simulink model "dds_cwg_32k"  (R2016b) (continued)
== system dds_cwg_32k/DDC_bu == (continued)
      mask help: FIR filter which can handle multiple time samples in parallel and decimates down to 1 time sample. If coefficients are symmetric, will automatically fold before multiplying.
      mask init:
      | dec_fir_async_init(gcb);
      settings: AttributesFormatString=|
      | 160 taps
      | 18_17 r/i
      mask TypeOptions quantization: Truncate | Round  (unbiased: +/- Inf) | Round  (unbiased: Even Values)
      mask TypeOptions adder_imp: Behavioral | Fabric | DSP48
      mask Callback bus_input:
      | onoff = get_param(gcb, 'bus_input');
      | set_param_state(gcb, 'input_width', onoff)
      | set_param_state(gcb, 'input_bp', onoff)
      | set_param_state(gcb, 'input_type', onoff)
      mask TypeOptions input_type: Signed | Unsigned
  edge_detect2 : Subsystem  AncestorBlock=casper_library_misc/edge_detect  mask(edge_detect){edge=Rising, polarity=Active High, x_in=[0 0.08 0.08 0.16 0.16 0.24 0.24 0.32 0.32 0.4], y_in=[-1 -1  1  1  1  1  1  1  1  1], x_out=[0.6 0.68 0.68 0.76 0.76 0.84 0.84 0.92 0.92 1], y_out=[-1 -1  1  1 -1 -1 -1 -1 -1 -1]}
      mask prompts: edge "Edge Type"; polarity "Output Polarity"; x_in "Input X Positions"; y_in "Input Y Positions"; x_out "Output X Positions"; y_out "Output Y Positions"
      mask help: Output is active for one-cycle after each edge of the specified type (i.e. rising, falling or both).
      mask init: same as dds_cwg_32k/edge_detect12
      settings: AttributesFormatString=|
      | rising edges
      | active high
      mask Help: Output is active for one-cycle after each edge of the specified type (i.e. rising, falling, or both).  Latency is zero.
      mask TypeOptions edge: Rising | Falling | Both
      mask TypeOptions polarity: Active High | Active Low
      mask Display:
      | plot(x_in_num,0.5+y_in_num*0.2);
      | plot(x_out_num,0.5+y_out_num*0.2);
  edge_detect3 : Subsystem  AncestorBlock=casper_library_misc/edge_detect  mask(edge_detect){edge=Rising, polarity=Active High, x_in=[0 0.08 0.08 0.16 0.16 0.24 0.24 0.32 0.32 0.4], y_in=[-1 -1  1  1  1  1  1  1  1  1], x_out=[0.6 0.68 0.68 0.76 0.76 0.84 0.84 0.92 0.92 1], y_out=[-1 -1  1  1 -1 -1 -1 -1 -1 -1]}
      mask prompts: edge "Edge Type"; polarity "Output Polarity"; x_in "Input X Positions"; y_in "Input Y Positions"; x_out "Output X Positions"; y_out "Output Y Positions"
      mask help: Output is active for one-cycle after each edge of the specified type (i.e. rising, falling or both).
      mask init: same as dds_cwg_32k/edge_detect12
      settings: AttributesFormatString=|
      | rising edges
      | active high
      mask Help: Output is active for one-cycle after each edge of the specified type (i.e. rising, falling, or both).  Latency is zero.
      mask TypeOptions edge: Rising | Falling | Both
      mask TypeOptions polarity: Active High | Active Low
      mask Display:
      | plot(x_in_num,0.5+y_in_num*0.2);
      | plot(x_out_num,0.5+y_out_num*0.2);
  mix_sync_cnt : Subsystem  Tag=xps:sw_reg  AncestorBlock=xps_library/Memory/software_register  mask(swreg){io_dir=To Processor, io_delay=0, init_val=0, sample_period=1, names=reg, bitwidths=8, bin_pts=0, arith_types=0, sim_port=off, show_format=on}
      mask prompts: io_dir "I/O direction"; io_delay "I/O delay"; init_val "Initial Value"; sample_period "Sample period"; names "Bitfield names [msb...lsb]"; bitwidths "Bitfield widths"; bin_pts "Bitfield binary pts"; arith_types "Bitfield types, ufix=0, fix=1, bool=2"; sim_port "Provide sim input/output?"; show_format "Print format string?"
      mask help: A 32-bit software-accessible register. Can be divided into bitfields of varying widths and types using the fields in the block mask.
      mask init:
      | swreg_init(gcb)
      settings: AttributesFormatString=1output:uf8.0 = 8 bits
      mask TypeOptions io_dir: To Processor | From Processor
      mask Callback io_dir:
      | mask_enables = get_param(gcb, 'MaskEnables');
      | mask_names = get_param(gcb, 'MaskNames');
      | if strcmp(get_param(gcb, 'io_dir'), 'To Processor'),
      |     mask_enables{ismember(mask_names, 'sample_period')} = 'off';
      | else
      |     mask_enables{ismember(mask_names, 'sample_period')} = 'on';
      | end
      | set_param(gcb, 'MaskEnables', mask_enables);
      mask DialogControl ParameterTabVar0 Prompt: Setup
  pipeline1 : Subsystem  AncestorBlock=casper_library_delays/pipeline  mask(pipeline){latency=2}
      mask help:
      | An explicitly laid-out delay line to avoid mapping to SRL16s
      | for use in pipelining to help achieve timing closure.
      mask init:
      | pipeline_init(gcb,'latency',latency);
      settings: AttributesFormatString=Latency = %<latency>
      mask Display:
      | port_label('input', 1, 'd');
      | port_label('output', 1, 'q');
      | disp(['Z^-', num2str(latency)]);
  pipeline10 : Subsystem  AncestorBlock=casper_library_delays/pipeline  mask(pipeline){latency=2}
      mask help:
      | An explicitly laid-out delay line to avoid mapping to SRL16s
      | for use in pipelining to help achieve timing closure.
      mask init:
      | pipeline_init(gcb,'latency',latency);
      settings: AttributesFormatString=Latency = %<latency>
      mask Display:
      | port_label('input', 1, 'd');
      | port_label('output', 1, 'q');
      | disp(['Z^-', num2str(latency)]);
  pipeline11 : Subsystem  AncestorBlock=casper_library_delays/pipeline  mask(pipeline){latency=2}
      mask help:
      | An explicitly laid-out delay line to avoid mapping to SRL16s
      | for use in pipelining to help achieve timing closure.
      mask init:
      | pipeline_init(gcb,'latency',latency);
      settings: AttributesFormatString=Latency = %<latency>
      mask Display:
      | port_label('input', 1, 'd');
      | port_label('output', 1, 'q');
      | disp(['Z^-', num2str(latency)]);
  pipeline12 : Subsystem  AncestorBlock=casper_library_delays/pipeline  mask(pipeline){latency=2}
      mask help:
      | An explicitly laid-out delay line to avoid mapping to SRL16s
      | for use in pipelining to help achieve timing closure.
      mask init:
      | pipeline_init(gcb,'latency',latency);
      settings: AttributesFormatString=Latency = %<latency>
      mask Display:
      | port_label('input', 1, 'd');
      | port_label('output', 1, 'q');
      | disp(['Z^-', num2str(latency)]);
  pipeline13 : Subsystem  AncestorBlock=casper_library_delays/pipeline  mask(pipeline){latency=2}
      mask help:
      | An explicitly laid-out delay line to avoid mapping to SRL16s
      | for use in pipelining to help achieve timing closure.
      mask init:
      | pipeline_init(gcb,'latency',latency);
      settings: AttributesFormatString=Latency = %<latency>
      mask Display:
      | port_label('input', 1, 'd');
      | port_label('output', 1, 'q');
      | disp(['Z^-', num2str(latency)]);
  pipeline14 : Subsystem  AncestorBlock=casper_library_delays/pipeline  mask(pipeline){latency=2}
      mask help:
      | An explicitly laid-out delay line to avoid mapping to SRL16s
      | for use in pipelining to help achieve timing closure.
      mask init:
      | pipeline_init(gcb,'latency',latency);
      settings: AttributesFormatString=Latency = %<latency>
      mask Display:
      | port_label('input', 1, 'd');
      | port_label('output', 1, 'q');
      | disp(['Z^-', num2str(latency)]);
  pipeline15 : Subsystem  AncestorBlock=casper_library_delays/pipeline  mask(pipeline){latency=2}
      mask help:
      | An explicitly laid-out delay line to avoid mapping to SRL16s
      | for use in pipelining to help achieve timing closure.
      mask init:
      | pipeline_init(gcb,'latency',latency);
      settings: AttributesFormatString=Latency = %<latency>
      mask Display:
      | port_label('input', 1, 'd');
      | port_label('output', 1, 'q');
      | disp(['Z^-', num2str(latency)]);
  pipeline16 : Subsystem  AncestorBlock=casper_library_delays/pipeline  mask(pipeline){latency=2}
      mask help:
      | An explicitly laid-out delay line to avoid mapping to SRL16s
      | for use in pipelining to help achieve timing closure.
      mask init:
      | pipeline_init(gcb,'latency',latency);
      settings: AttributesFormatString=Latency = %<latency>
      mask Display:
      | port_label('input', 1, 'd');
      | port_label('output', 1, 'q');
      | disp(['Z^-', num2str(latency)]);
  pipeline17 : Subsystem  AncestorBlock=casper_library_delays/pipeline  mask(pipeline){latency=2}
      mask help:
      | An explicitly laid-out delay line to avoid mapping to SRL16s
      | for use in pipelining to help achieve timing closure.
      mask init:
      | pipeline_init(gcb,'latency',latency);
      settings: AttributesFormatString=Latency = %<latency>
      mask Display:
      | port_label('input', 1, 'd');
      | port_label('output', 1, 'q');
      | disp(['Z^-', num2str(latency)]);
  pipeline18 : Subsystem  Commented=on  AncestorBlock=casper_library_delays/pipeline  mask(pipeline){latency=2}
      mask help:
      | An explicitly laid-out delay line to avoid mapping to SRL16s
      | for use in pipelining to help achieve timing closure.
      mask init:
      | pipeline_init(gcb,'latency',latency);
      settings: AttributesFormatString=Latency = %<latency>
      mask Display:
      | port_label('input', 1, 'd');
      | port_label('output', 1, 'q');
      | disp(['Z^-', num2str(latency)]);
  pipeline19 : Subsystem  AncestorBlock=casper_library_delays/pipeline  mask(pipeline){latency=2}
      mask help:
      | An explicitly laid-out delay line to avoid mapping to SRL16s
      | for use in pipelining to help achieve timing closure.
      mask init:
      | pipeline_init(gcb,'latency',latency);
      settings: AttributesFormatString=Latency = %<latency>
      mask Display:
      | port_label('input', 1, 'd');
      | port_label('output', 1, 'q');
      | disp(['Z^-', num2str(latency)]);
  pipeline2 : Subsystem  AncestorBlock=casper_library_delays/pipeline  mask(pipeline){latency=2}
      mask help:
      | An explicitly laid-out delay line to avoid mapping to SRL16s
      | for use in pipelining to help achieve timing closure.
      mask init:
      | pipeline_init(gcb,'latency',latency);
      settings: AttributesFormatString=Latency = %<latency>
      mask Display:
      | port_label('input', 1, 'd');
      | port_label('output', 1, 'q');
      | disp(['Z^-', num2str(latency)]);
  pipeline20 : Subsystem  AncestorBlock=casper_library_delays/pipeline  mask(pipeline){latency=2}
      mask help:
      | An explicitly laid-out delay line to avoid mapping to SRL16s
      | for use in pipelining to help achieve timing closure.
      mask init:
      | pipeline_init(gcb,'latency',latency);
      settings: AttributesFormatString=Latency = %<latency>
      mask Display:
      | port_label('input', 1, 'd');
      | port_label('output', 1, 'q');
      | disp(['Z^-', num2str(latency)]);
  pipeline21 : Subsystem  AncestorBlock=casper_library_delays/pipeline  mask(pipeline){latency=2}
      mask help:
      | An explicitly laid-out delay line to avoid mapping to SRL16s
      | for use in pipelining to help achieve timing closure.
      mask init:
      | pipeline_init(gcb,'latency',latency);
      settings: AttributesFormatString=Latency = %<latency>
      mask Display:
      | port_label('input', 1, 'd');
      | port_label('output', 1, 'q');
      | disp(['Z^-', num2str(latency)]);
  pipeline22 : Subsystem  AncestorBlock=casper_library_delays/pipeline  mask(pipeline){latency=2}
      mask help:
      | An explicitly laid-out delay line to avoid mapping to SRL16s
      | for use in pipelining to help achieve timing closure.
      mask init:
      | pipeline_init(gcb,'latency',latency);
      settings: AttributesFormatString=Latency = %<latency>
      mask Display:
      | port_label('input', 1, 'd');
      | port_label('output', 1, 'q');
      | disp(['Z^-', num2str(latency)]);
  pipeline229 : Subsystem  AncestorBlock=casper_library_delays/pipeline  mask(pipeline){latency=2}
      mask help:
      | An explicitly laid-out delay line to avoid mapping to SRL16s
      | for use in pipelining to help achieve timing closure.
      mask init:
      | pipeline_init(gcb,'latency',latency);
      settings: AttributesFormatString=Latency = %<latency>
      mask Display:
      | port_label('input', 1, 'd');
      | port_label('output', 1, 'q');
      | disp(['Z^-', num2str(latency)]);
  pipeline23 : Subsystem  AncestorBlock=casper_library_delays/pipeline  mask(pipeline){latency=1}
      mask help:
      | An explicitly laid-out delay line to avoid mapping to SRL16s
      | for use in pipelining to help achieve timing closure.
      mask init:
      | pipeline_init(gcb,'latency',latency);
      settings: AttributesFormatString=Latency = %<latency>
      mask Display:
      | port_label('input', 1, 'd');
      | port_label('output', 1, 'q');
      | disp(['Z^-', num2str(latency)]);
  pipeline230 : Subsystem  AncestorBlock=casper_library_delays/pipeline  mask(pipeline){latency=2}
      mask help:
      | An explicitly laid-out delay line to avoid mapping to SRL16s
      | for use in pipelining to help achieve timing closure.
      mask init:
      | pipeline_init(gcb,'latency',latency);
      settings: AttributesFormatString=Latency = %<latency>
      mask Display:
      | port_label('input', 1, 'd');
      | port_label('output', 1, 'q');
      | disp(['Z^-', num2str(latency)]);
  pipeline24 : Subsystem  AncestorBlock=casper_library_delays/pipeline  mask(pipeline){latency=1}
      mask help:
      | An explicitly laid-out delay line to avoid mapping to SRL16s
      | for use in pipelining to help achieve timing closure.
      mask init:
      | pipeline_init(gcb,'latency',latency);
      settings: AttributesFormatString=Latency = %<latency>
      mask Display:
      | port_label('input', 1, 'd');
      | port_label('output', 1, 'q');
      | disp(['Z^-', num2str(latency)]);
  pipeline25 : Subsystem  AncestorBlock=casper_library_delays/pipeline  mask(pipeline){latency=2}
      mask help:
      | An explicitly laid-out delay line to avoid mapping to SRL16s
      | for use in pipelining to help achieve timing closure.
      mask init:
      | pipeline_init(gcb,'latency',latency);
      settings: AttributesFormatString=Latency = %<latency>
      mask Display:
      | port_label('input', 1, 'd');
      | port_label('output', 1, 'q');
      | disp(['Z^-', num2str(latency)]);
  pipeline26 : Subsystem  AncestorBlock=casper_library_delays/pipeline  mask(pipeline){latency=2}
      mask help:
      | An explicitly laid-out delay line to avoid mapping to SRL16s
      | for use in pipelining to help achieve timing closure.
      mask init:
      | pipeline_init(gcb,'latency',latency);
      settings: AttributesFormatString=Latency = %<latency>
      mask Display:
      | port_label('input', 1, 'd');
      | port_label('output', 1, 'q');
      | disp(['Z^-', num2str(latency)]);
  pipeline27 : Subsystem  Commented=on  AncestorBlock=casper_library_delays/pipeline  mask(pipeline){latency=2}
      mask help:
      | An explicitly laid-out delay line to avoid mapping to SRL16s
      | for use in pipelining to help achieve timing closure.
      mask init:
      | pipeline_init(gcb,'latency',latency);
      settings: AttributesFormatString=Latency = %<latency>
      mask Display:
      | port_label('input', 1, 'd');
      | port_label('output', 1, 'q');
      | disp(['Z^-', num2str(latency)]);
  pipeline28 : Subsystem  Commented=on  AncestorBlock=casper_library_delays/pipeline  mask(pipeline){latency=1}
      mask help:
      | An explicitly laid-out delay line to avoid mapping to SRL16s
      | for use in pipelining to help achieve timing closure.
      mask init:
      | pipeline_init(gcb,'latency',latency);
      settings: AttributesFormatString=Latency = %<latency>
      mask Display:
      | port_label('input', 1, 'd');
      | port_label('output', 1, 'q');
      | disp(['Z^-', num2str(latency)]);
  pipeline29 : Subsystem  AncestorBlock=casper_library_delays/pipeline  mask(pipeline){latency=2}
      mask help:
      | An explicitly laid-out delay line to avoid mapping to SRL16s
      | for use in pipelining to help achieve timing closure.
      mask init:
      | pipeline_init(gcb,'latency',latency);
      settings: AttributesFormatString=Latency = %<latency>
      mask Display:
      | port_label('input', 1, 'd');
      | port_label('output', 1, 'q');
      | disp(['Z^-', num2str(latency)]);
  pipeline3 : Subsystem  AncestorBlock=casper_library_delays/pipeline  mask(pipeline){latency=2}
      mask help:
      | An explicitly laid-out delay line to avoid mapping to SRL16s
      | for use in pipelining to help achieve timing closure.
      mask init:
      | pipeline_init(gcb,'latency',latency);
      settings: AttributesFormatString=Latency = %<latency>
      mask Display:
      | port_label('input', 1, 'd');
      | port_label('output', 1, 'q');
      | disp(['Z^-', num2str(latency)]);
  pipeline30 : Subsystem  AncestorBlock=casper_library_delays/pipeline  mask(pipeline){latency=2}
      mask help:
      | An explicitly laid-out delay line to avoid mapping to SRL16s
      | for use in pipelining to help achieve timing closure.
      mask init:
      | pipeline_init(gcb,'latency',latency);
      settings: AttributesFormatString=Latency = %<latency>
      mask Display:
      | port_label('input', 1, 'd');
      | port_label('output', 1, 'q');
      | disp(['Z^-', num2str(latency)]);
  pipeline31 : Subsystem  AncestorBlock=casper_library_delays/pipeline  mask(pipeline){latency=2}
      mask help:
      | An explicitly laid-out delay line to avoid mapping to SRL16s
      | for use in pipelining to help achieve timing closure.
      mask init:
      | pipeline_init(gcb,'latency',latency);
      settings: AttributesFormatString=Latency = %<latency>
      mask Display:
      | port_label('input', 1, 'd');
      | port_label('output', 1, 'q');
      | disp(['Z^-', num2str(latency)]);
  pipeline32 : Subsystem  Commented=on  AncestorBlock=casper_library_delays/pipeline  mask(pipeline){latency=2}
      mask help:
      | An explicitly laid-out delay line to avoid mapping to SRL16s
      | for use in pipelining to help achieve timing closure.
      mask init:
      | pipeline_init(gcb,'latency',latency);
      settings: AttributesFormatString=Latency = %<latency>
      mask Display:
      | port_label('input', 1, 'd');
      | port_label('output', 1, 'q');
      | disp(['Z^-', num2str(latency)]);
  pipeline33 : Subsystem  Commented=on  AncestorBlock=casper_library_delays/pipeline  mask(pipeline){latency=2}
      mask help:
      | An explicitly laid-out delay line to avoid mapping to SRL16s
      | for use in pipelining to help achieve timing closure.
      mask init:
      | pipeline_init(gcb,'latency',latency);
      settings: AttributesFormatString=Latency = %<latency>
      mask Display:
      | port_label('input', 1, 'd');
      | port_label('output', 1, 'q');
      | disp(['Z^-', num2str(latency)]);
  pipeline34 : Subsystem  AncestorBlock=casper_library_delays/pipeline  mask(pipeline){latency=2}
      mask help:
      | An explicitly laid-out delay line to avoid mapping to SRL16s
      | for use in pipelining to help achieve timing closure.
      mask init:
      | pipeline_init(gcb,'latency',latency);
      settings: AttributesFormatString=Latency = %<latency>
      mask Display:
      | port_label('input', 1, 'd');
      | port_label('output', 1, 'q');
      | disp(['Z^-', num2str(latency)]);
  pipeline35 : Subsystem  AncestorBlock=casper_library_delays/pipeline  mask(pipeline){latency=1}
      mask help:
      | An explicitly laid-out delay line to avoid mapping to SRL16s
      | for use in pipelining to help achieve timing closure.
      mask init:
      | pipeline_init(gcb,'latency',latency);
      settings: AttributesFormatString=Latency = %<latency>
      mask Display:
      | port_label('input', 1, 'd');
      | port_label('output', 1, 'q');
      | disp(['Z^-', num2str(latency)]);
  pipeline36 : Subsystem  AncestorBlock=casper_library_delays/pipeline  mask(pipeline){latency=2}
      mask help:
      | An explicitly laid-out delay line to avoid mapping to SRL16s
      | for use in pipelining to help achieve timing closure.
      mask init:
      | pipeline_init(gcb,'latency',latency);
      settings: AttributesFormatString=Latency = %<latency>
      mask Display:
      | port_label('input', 1, 'd');
      | port_label('output', 1, 'q');
      | disp(['Z^-', num2str(latency)]);
  pipeline37 : Subsystem  AncestorBlock=casper_library_delays/pipeline  mask(pipeline){latency=2}
      mask help:
      | An explicitly laid-out delay line to avoid mapping to SRL16s
      | for use in pipelining to help achieve timing closure.
      mask init:
      | pipeline_init(gcb,'latency',latency);
      settings: AttributesFormatString=Latency = %<latency>
      mask Display:
      | port_label('input', 1, 'd');
      | port_label('output', 1, 'q');
      | disp(['Z^-', num2str(latency)]);
  pipeline38 : Subsystem  AncestorBlock=casper_library_delays/pipeline  mask(pipeline){latency=2}
      mask help:
      | An explicitly laid-out delay line to avoid mapping to SRL16s
      | for use in pipelining to help achieve timing closure.
      mask init:
      | pipeline_init(gcb,'latency',latency);
      settings: AttributesFormatString=Latency = %<latency>
      mask Display:
      | port_label('input', 1, 'd');
      | port_label('output', 1, 'q');
      | disp(['Z^-', num2str(latency)]);
  pipeline39 : Subsystem  AncestorBlock=casper_library_delays/pipeline  mask(pipeline){latency=2}
      mask help:
      | An explicitly laid-out delay line to avoid mapping to SRL16s
      | for use in pipelining to help achieve timing closure.
      mask init:
      | pipeline_init(gcb,'latency',latency);
      settings: AttributesFormatString=Latency = %<latency>
      mask Display:
      | port_label('input', 1, 'd');
      | port_label('output', 1, 'q');
      | disp(['Z^-', num2str(latency)]);
  pipeline4 : Subsystem  AncestorBlock=casper_library_delays/pipeline  mask(pipeline){latency=2}
      mask help:
      | An explicitly laid-out delay line to avoid mapping to SRL16s
      | for use in pipelining to help achieve timing closure.
      mask init:
      | pipeline_init(gcb,'latency',latency);
      settings: AttributesFormatString=Latency = %<latency>
      mask Display:
      | port_label('input', 1, 'd');
      | port_label('output', 1, 'q');
      | disp(['Z^-', num2str(latency)]);
  pipeline40 : Subsystem  AncestorBlock=casper_library_delays/pipeline  mask(pipeline){latency=2}
      mask help:
      | An explicitly laid-out delay line to avoid mapping to SRL16s
      | for use in pipelining to help achieve timing closure.
      mask init:
      | pipeline_init(gcb,'latency',latency);
      settings: AttributesFormatString=Latency = %<latency>
      mask Display:
      | port_label('input', 1, 'd');
      | port_label('output', 1, 'q');
      | disp(['Z^-', num2str(latency)]);
  pipeline41 : Subsystem  AncestorBlock=casper_library_delays/pipeline  mask(pipeline){latency=2}
      mask help:
      | An explicitly laid-out delay line to avoid mapping to SRL16s
      | for use in pipelining to help achieve timing closure.
      mask init:
      | pipeline_init(gcb,'latency',latency);
      settings: AttributesFormatString=Latency = %<latency>
      mask Display:
      | port_label('input', 1, 'd');
      | port_label('output', 1, 'q');
      | disp(['Z^-', num2str(latency)]);
  pipeline42 : Subsystem  Commented=on  AncestorBlock=casper_library_delays/pipeline  mask(pipeline){latency=2}
      mask help:
      | An explicitly laid-out delay line to avoid mapping to SRL16s
      | for use in pipelining to help achieve timing closure.
      mask init:
      | pipeline_init(gcb,'latency',latency);
      settings: AttributesFormatString=Latency = %<latency>
      mask Display:
      | port_label('input', 1, 'd');
      | port_label('output', 1, 'q');
      | disp(['Z^-', num2str(latency)]);
  pipeline7 : Subsystem  AncestorBlock=casper_library_delays/pipeline  mask(pipeline){latency=2}
      mask help:
      | An explicitly laid-out delay line to avoid mapping to SRL16s
      | for use in pipelining to help achieve timing closure.
      mask init:
      | pipeline_init(gcb,'latency',latency);
      settings: AttributesFormatString=Latency = %<latency>
      mask Display:
      | port_label('input', 1, 'd');
      | port_label('output', 1, 'q');
      | disp(['Z^-', num2str(latency)]);
  pipeline8 : Subsystem  AncestorBlock=casper_library_delays/pipeline  mask(pipeline){latency=2}
      mask help:
      | An explicitly laid-out delay line to avoid mapping to SRL16s
      | for use in pipelining to help achieve timing closure.
      mask init:
      | pipeline_init(gcb,'latency',latency);
      settings: AttributesFormatString=Latency = %<latency>
      mask Display:
      | port_label('input', 1, 'd');
      | port_label('output', 1, 'q');
      | disp(['Z^-', num2str(latency)]);
  pipeline9 : Subsystem  AncestorBlock=casper_library_delays/pipeline  mask(pipeline){latency=2}
      mask help:
      | An explicitly laid-out delay line to avoid mapping to SRL16s
      | for use in pipelining to help achieve timing closure.
      mask init:
      | pipeline_init(gcb,'latency',latency);
      settings: AttributesFormatString=Latency = %<latency>
      mask Display:
      | port_label('input', 1, 'd');
      | port_label('output', 1, 'q');
      | disp(['Z^-', num2str(latency)]);
  snap_ddc : Subsystem  Tag=casper:bitsnap  AncestorBlock=casper_library_scopes/bitfield_snapshot  mask(bitsnap){io_names=[p0_re p0_im dv sync], io_widths=[18 18 1 1], io_bps=[17 17 0 0], io_types=[1 1 2 2], show_format=on, snap_storage=bram, snap_dram_dimm=2, snap_dram_clock=250, snap_nsamples=15, snap_data_width=64, snap_offset=off, snap_circap=off, snap_value=off, snap_ext_arm=off, snap_ext_circ=off, snap_provide_outputs=on, snap_use_dsp48=on, snap_delay=2, extra_names=timestamp, extra_widths=32, extra_bps=0, extra_types=0}
      mask prompts: io_names "Names [msb...lsb]"; io_widths "Bitwidths [msb...lsb]"; io_bps "Binary pts [msb...lsb]"; io_types "Data types [msb...lsb], ufix=0, fix=1, bool=2"; show_format "Print format string?"; snap_storage "Storage medium"; snap_dram_dimm "DRAM dimm"; snap_dram_clock "DRAM clock rate"; snap_nsamples "Number of Samples (2^?)"; snap_data_width "Data width"; snap_offset "Start delay support"; snap_circap "Circular capture support"; snap_value "Extra value capture support"; snap_ext_arm "External arm support"; snap_ext_circ "External circular capture support"; snap_provide_outputs "Provide outputs"; snap_use_dsp48 "Use DSP48s to implement counters"; snap_delay "Pre-snapshot delay"; extra_names "Names [msb...lsb]"; extra_widths "Widths [msb...lsb]"; extra_bps "Binary pts [msb...lsb]"; extra_types "Data types [msb...lsb], ufix=0, fix=1, bool=2"
      mask help:
      | A wrapped snapshot block. Allows specification of inputs to a snapblock word so the toolflow can automatically set up the scope.
      | See documentation for snapshot for explanation of parameters.
      mask init:
      | bitsnap_init(gcb);
      settings: AttributesFormatString=|
      | snap(4):f18.17,f18.17,b,b = 38 bits\n64 wide, 32768 deep
      | debugID: 2990984
      mask TypeOptions snap_storage: bram | dram
      mask Callback snap_storage: bitsnap_callback();
      mask TypeOptions snap_dram_dimm: 1 | 2 | 3 | 4
      mask TypeOptions snap_dram_clock: 200 | 250 | 266 | 300 | 333
      mask TypeOptions snap_data_width: 8 | 16 | 32 | 64 | 128
      mask Callback snap_value: bitsnap_callback();
      mask Callback snap_ext_arm: bitsnap_callback();
      mask Callback snap_ext_circ: bitsnap_callback();
      mask Callback snap_provide_outputs: bitsnap_callback();
  snap_ddc1 : Subsystem  Tag=casper:bitsnap  Commented=on  AncestorBlock=casper_library_scopes/bitfield_snapshot  mask(bitsnap){io_names=[p0_re p0_im dv sync], io_widths=[18 18 1 1], io_bps=[17 17 0 0], io_types=[1 1 2 2], show_format=on, snap_storage=bram, snap_dram_dimm=2, snap_dram_clock=250, snap_nsamples=12, snap_data_width=64, snap_offset=off, snap_circap=off, snap_value=off, snap_ext_arm=off, snap_ext_circ=off, snap_provide_outputs=on, snap_use_dsp48=on, snap_delay=2, extra_names=timestamp, extra_widths=32, extra_bps=0, extra_types=0}
      mask prompts: io_names "Names [msb...lsb]"; io_widths "Bitwidths [msb...lsb]"; io_bps "Binary pts [msb...lsb]"; io_types "Data types [msb...lsb], ufix=0, fix=1, bool=2"; show_format "Print format string?"; snap_storage "Storage medium"; snap_dram_dimm "DRAM dimm"; snap_dram_clock "DRAM clock rate"; snap_nsamples "Number of Samples (2^?)"; snap_data_width "Data width"; snap_offset "Start delay support"; snap_circap "Circular capture support"; snap_value "Extra value capture support"; snap_ext_arm "External arm support"; snap_ext_circ "External circular capture support"; snap_provide_outputs "Provide outputs"; snap_use_dsp48 "Use DSP48s to implement counters"; snap_delay "Pre-snapshot delay"; extra_names "Names [msb...lsb]"; extra_widths "Widths [msb...lsb]"; extra_bps "Binary pts [msb...lsb]"; extra_types "Data types [msb...lsb], ufix=0, fix=1, bool=2"
      mask help:
      | A wrapped snapshot block. Allows specification of inputs to a snapblock word so the toolflow can automatically set up the scope.
      | See documentation for snapshot for explanation of parameters.
      mask init:
      | bitsnap_init(gcb);
      settings: AttributesFormatString=|
      | snap(4):f18.17,f18.17,b,b = 38 bits\n64 wide, 4096 deep
      | debugID: 92714853
      mask TypeOptions snap_storage: bram | dram
      mask Callback snap_storage: bitsnap_callback();
      mask TypeOptions snap_dram_dimm: 1 | 2 | 3 | 4
      mask TypeOptions snap_dram_clock: 200 | 250 | 266 | 300 | 333
      mask TypeOptions snap_data_width: 8 | 16 | 32 | 64 | 128
      mask Callback snap_value: bitsnap_callback();
      mask Callback snap_ext_arm: bitsnap_callback();
      mask Callback snap_ext_circ: bitsnap_callback();
      mask Callback snap_provide_outputs: bitsnap_callback();
  snap_mix_im : Subsystem  Tag=casper:bitsnap  AncestorBlock=casper_library_scopes/bitfield_snapshot  mask(bitsnap){io_names=[p0_d0 p0_d1 p0_d2 p0_d3 dv sync dec_dv dec_sync], io_widths=[18 18 18 18 1 1 1 1], io_bps=[17 17 17 17 0 0 0 0], io_types=[1 1 1 1 2 2 2 2], show_format=on, snap_storage=bram, snap_dram_dimm=2, snap_dram_clock=250, snap_nsamples=12, snap_data_width=128, snap_offset=off, snap_circap=off, snap_value=off, snap_ext_arm=off, snap_ext_circ=off, snap_provide_outputs=on, snap_use_dsp48=on, snap_delay=2, extra_names=timestamp, extra_widths=32, extra_bps=0, extra_types=0}
      mask prompts: io_names "Names [msb...lsb]"; io_widths "Bitwidths [msb...lsb]"; io_bps "Binary pts [msb...lsb]"; io_types "Data types [msb...lsb], ufix=0, fix=1, bool=2"; show_format "Print format string?"; snap_storage "Storage medium"; snap_dram_dimm "DRAM dimm"; snap_dram_clock "DRAM clock rate"; snap_nsamples "Number of Samples (2^?)"; snap_data_width "Data width"; snap_offset "Start delay support"; snap_circap "Circular capture support"; snap_value "Extra value capture support"; snap_ext_arm "External arm support"; snap_ext_circ "External circular capture support"; snap_provide_outputs "Provide outputs"; snap_use_dsp48 "Use DSP48s to implement counters"; snap_delay "Pre-snapshot delay"; extra_names "Names [msb...lsb]"; extra_widths "Widths [msb...lsb]"; extra_bps "Binary pts [msb...lsb]"; extra_types "Data types [msb...lsb], ufix=0, fix=1, bool=2"
      mask help:
      | A wrapped snapshot block. Allows specification of inputs to a snapblock word so the toolflow can automatically set up the scope.
      | See documentation for snapshot for explanation of parameters.
      mask init:
      | bitsnap_init(gcb);
      settings: AttributesFormatString=|
      | snap(8):f18.17,f18.17,f18.17,f18.17,b,b,b,b = 76 bits\n128 wide, 4096 deep
      | debugID: 85174924
      mask TypeOptions snap_storage: bram | dram
      mask Callback snap_storage: bitsnap_callback();
      mask TypeOptions snap_dram_dimm: 1 | 2 | 3 | 4
      mask TypeOptions snap_dram_clock: 200 | 250 | 266 | 300 | 333
      mask TypeOptions snap_data_width: 8 | 16 | 32 | 64 | 128
      mask Callback snap_value: bitsnap_callback();
      mask Callback snap_ext_arm: bitsnap_callback();
      mask Callback snap_ext_circ: bitsnap_callback();
      mask Callback snap_provide_outputs: bitsnap_callback();
  snap_mix_re : Subsystem  Tag=casper:bitsnap  AncestorBlock=casper_library_scopes/bitfield_snapshot  mask(bitsnap){io_names=[p0_d0 p0_d1 p0_d2 p0_d3 dv sync dec_dv dec_sync], io_widths=[18 18 18 18 1 1 1 1], io_bps=[17 17 17 17 0 0 0 0], io_types=[1 1 1 1 2 2 2 2], show_format=on, snap_storage=bram, snap_dram_dimm=2, snap_dram_clock=250, snap_nsamples=12, snap_data_width=128, snap_offset=off, snap_circap=off, snap_value=off, snap_ext_arm=off, snap_ext_circ=off, snap_provide_outputs=on, snap_use_dsp48=on, snap_delay=2, extra_names=timestamp, extra_widths=32, extra_bps=0, extra_types=0}
      mask prompts: io_names "Names [msb...lsb]"; io_widths "Bitwidths [msb...lsb]"; io_bps "Binary pts [msb...lsb]"; io_types "Data types [msb...lsb], ufix=0, fix=1, bool=2"; show_format "Print format string?"; snap_storage "Storage medium"; snap_dram_dimm "DRAM dimm"; snap_dram_clock "DRAM clock rate"; snap_nsamples "Number of Samples (2^?)"; snap_data_width "Data width"; snap_offset "Start delay support"; snap_circap "Circular capture support"; snap_value "Extra value capture support"; snap_ext_arm "External arm support"; snap_ext_circ "External circular capture support"; snap_provide_outputs "Provide outputs"; snap_use_dsp48 "Use DSP48s to implement counters"; snap_delay "Pre-snapshot delay"; extra_names "Names [msb...lsb]"; extra_widths "Widths [msb...lsb]"; extra_bps "Binary pts [msb...lsb]"; extra_types "Data types [msb...lsb], ufix=0, fix=1, bool=2"
      mask help:
      | A wrapped snapshot block. Allows specification of inputs to a snapblock word so the toolflow can automatically set up the scope.
      | See documentation for snapshot for explanation of parameters.
      mask init:
      | bitsnap_init(gcb);
      settings: AttributesFormatString=|
      | snap(8):f18.17,f18.17,f18.17,f18.17,b,b,b,b = 76 bits\n128 wide, 4096 deep
      | debugID: 93248285
      mask TypeOptions snap_storage: bram | dram
      mask Callback snap_storage: bitsnap_callback();
      mask TypeOptions snap_dram_dimm: 1 | 2 | 3 | 4
      mask TypeOptions snap_dram_clock: 200 | 250 | 266 | 300 | 333
      mask TypeOptions snap_data_width: 8 | 16 | 32 | 64 | 128
      mask Callback snap_value: bitsnap_callback();
      mask Callback snap_ext_arm: bitsnap_callback();
      mask Callback snap_ext_circ: bitsnap_callback();
      mask Callback snap_provide_outputs: bitsnap_callback();
  ddc_sync : Outport
  ddc_pol0 : Outport  Port=2
  ddc_pol1 : Outport  Port=3
  ddc_dv : Outport  Port=4
  ddc_of : Outport  Port=5
  tag scope: dec_d80_0=global, dec_d80_1=global, dec_dv=global, dec_sync=global, mix_80_0=global, mix_dvalid=global, mix_sync=global
  wires:
  Delay7/1 -> [ddc_dvalid]
  bus_expand14/1(msb_out16) -> Gateway Out42/1
  Gateway Out42/1 -> To Workspace42/1
  bus_expand13/1(msb_out16) -> Gateway Out41/1
  Gateway Out41/1 -> To Workspace41/1
  Register4/1 -> [dec_d80_1]
  [mix_80_1] -> Register4/1
  Gateway Out40/1 -> To Workspace40/1
  [mix_dvalid] -> Register1/3, Logical1/2, Gateway Out40/1, Gateway Out39/1, Register3/3, Register4/3
  [mix_sync] -> Register3/2, Register4/2
  Register3/1 -> [dec_d80_0], bus_expand13/1(bus_in)
  [mix_80_0] -> Register3/1, bus_expand14/1(bus_in)
  Logical1/1 -> edge_detect3/1(in), Gateway Out37/1, Logical2/2, Gateway Out36/1
  Gateway Out39/1 -> To Workspace39/1
  Gateway Out38/1 -> To Workspace38/1
  Gateway Out37/1 -> To Workspace37/1
  Gateway Out36/1 -> To Workspace36/1
  Gateway Out35/1 -> To Workspace35/1
  Gateway Out34/1 -> To Workspace34/1
  Gateway Out33/1 -> To Workspace33/1
  Logical2/1 -> edge_detect2/1(in), Register2/2, Gateway Out33/1
  Register2/1 -> Logical2/1, Gateway Out34/1
  [mix_sync] -> Register2/1, Gateway Out35/1, Register2/3, Register1/2
  Assert/1 -> Register1/1
  Inverter/1 -> Assert/1
  edge_detect2/1(out) -> [dec_sync]
  edge_detect3/1(out) -> [dec_dv]
  Register1/1 -> Inverter/1, Logical1/1, Gateway Out38/1
  Delay8/1 -> [mix_80_1]
  Delay2/1 -> [mix_dvalid]
  Delay1/1 -> [mix_80_0]
  dec_fir_async3/1(sync_out) -> [ddc_sync]
  bus_mult1/1(a*b) -> Delay8/1
  bus_expand/2(lsb_out1) -> Delay2/1
  bus_mult/1(a*b) -> Delay1/1
  Delay/1 -> [ddc_pol0], [ddc_pol1], Gateway Out22/1
  [dec_d80_0] -> Delay3/1, bus_expand6/1(bus_in)
  bus_expand11/4(out13) -> Gateway Out32/1
  Gateway Out32/1 -> To Workspace31/1
  bus_expand11/3(out14) -> Gateway Out31/1
  Gateway Out31/1 -> To Workspace30/1
  bus_expand11/2(out15) -> Gateway Out30/1
  bus_expand11/1(msb_out16) -> Gateway Out29/1
  Gateway Out30/1 -> To Workspace32/1
  Gateway Out29/1 -> To Workspace28/1
  bus_expand12/2(out15) -> Gateway Out28/1
  bus_expand12/1(msb_out16) -> Gateway Out26/1
  Gateway Out28/1 -> To Workspace29/1
  Gateway Out26/1 -> To Workspace26/1
  pipeline4/1(q) -> snap_mix_im/8(in_dec_sync)
  pipeline3/1(q) -> snap_mix_im/7(in_dec_dv)
  pipeline31/1(q) -> snap_mix_im/6(in_sync)
  pipeline30/1(q) -> snap_mix_im/5(in_dv)
  pipeline34/1(q) -> snap_mix_im/9(we)
  pipeline35/1(q) -> snap_mix_im/10(trig)
  dec_fir_async3/2(dout) -> Delay/1
  dec_fir_async1/2(dout) -> bus_expand7/1(bus_in), bus_expand8/1(bus_in)
  bus_expand8/2(lsb_out1) -> pipeline18/1(d)
  bus_expand8/1(msb_out2) -> pipeline27/1(d)
  pipeline42/1(q) -> snap_ddc1/5(we)
  pipeline28/1(q) -> snap_ddc1/6(trig)
  pipeline33/1(q) -> snap_ddc1/4(in_sync)
  pipeline32/1(q) -> snap_ddc1/3(in_dv)
  pipeline18/1(q) -> snap_ddc1/2(in_p0_im)
  pipeline27/1(q) -> snap_ddc1/1(in_p0_re)
  Delay4/1 -> Gateway Out8/1, pipeline28/1(d), pipeline33/1(d)
  Delay6/1 -> Gateway Out6/1, pipeline42/1(d), pipeline32/1(d)
  dec_fir_async3/3(dv_out) -> Delay7/1
  pipeline23/1(q) -> snap_mix_re/10(trig)
  pipeline19/1(q) -> snap_mix_re/9(we)
  pipeline17/1(q) -> snap_mix_re/8(in_dec_sync)
  pipeline41/1(q) -> snap_mix_re/7(in_dec_dv)
  pipeline16/1(q) -> snap_mix_re/6(in_sync)
  pipeline15/1(q) -> snap_mix_re/5(in_dv)
  pipeline10/1(q) -> snap_mix_re/4(in_p0_d3)
  pipeline9/1(q) -> snap_mix_re/3(in_p0_d2)
  pipeline8/1(q) -> snap_mix_re/2(in_p0_d1)
  pipeline7/1(q) -> snap_mix_re/1(in_p0_d0)
  Constant/1 -> [ddc_of_pol1], [ddc_of_pol0]
  Gateway Out22/1 -> To Workspace22/1
  Gateway Out8/1 -> To Workspace21/1
  dec_fir_async1/1(sync_out) -> Delay4/1
  dec_fir_async1/3(dv_out) -> Delay6/1
  Gateway Out6/1 -> To Workspace19/1
  Concat1/1 -> ddc_of/1
  [ddc_of_pol1] -> Concat1/2
  [ddc_of_pol0] -> Concat1/1
  Gateway Out24/1 -> To Workspace24/1
  Gateway Out7/1 -> To Workspace20/1
  [dec_sync] -> pipeline17/1(d), pipeline4/1(d)
  [dec_dv] -> pipeline41/1(d), pipeline3/1(d)
  Gateway Out3/1 -> To Workspace11/1
  From1/1 -> bus_expand10/1(bus_in), Delay5/1
  Gateway Out23/1 -> To Workspace23/1
  From15/1 -> dec_fir_async2/1(sync_in)
  From26/1 -> dec_fir_async2/2(dv_in)
  [dec_sync] -> Gateway Out23/1, dec_fir_async3/1(sync_in)
  [dec_sync] -> pipeline39/1(d)
  pipeline40/1(q) -> Counter3/1
  pipeline39/1(q) -> Counter3/2
  Counter3/1 -> ddc_in_sync_cnt/1(out_reg)
  [cnt_rst] -> pipeline40/1(d)
  [dec_dv] -> Gateway Out3/1, dec_fir_async3/2(dv_in)
  bus_expand10/16(lsb_out1) -> dec_fir_async2/34(imag16)
  bus_expand10/15(out2) -> dec_fir_async2/33(real16)
  bus_expand10/13(out4) -> dec_fir_async2/31(real15)
  bus_expand10/14(out3) -> dec_fir_async2/32(imag15)
  bus_expand10/12(out5) -> dec_fir_async2/30(imag14)
  bus_expand10/11(out6) -> dec_fir_async2/29(real14)
  bus_expand10/10(out7) -> dec_fir_async2/28(imag13)
  bus_expand10/9(out8) -> dec_fir_async2/27(real13)
  bus_expand10/8(out9) -> dec_fir_async2/26(imag12)
  bus_expand10/6(out11) -> dec_fir_async2/24(imag11)
  bus_expand10/7(out10) -> dec_fir_async2/25(real12)
  bus_expand10/5(out12) -> dec_fir_async2/23(real11)
  bus_expand10/4(out13) -> dec_fir_async2/22(imag10)
  bus_expand10/2(out15) -> dec_fir_async2/20(imag9)
  bus_expand10/3(out14) -> dec_fir_async2/21(real10)
  bus_expand10/1(msb_out16) -> dec_fir_async2/19(real9)
  bus_expand9/16(lsb_out1) -> dec_fir_async2/18(imag8)
  bus_expand9/15(out2) -> dec_fir_async2/17(real8)
  bus_expand9/14(out3) -> dec_fir_async2/16(imag7)
  bus_expand9/13(out4) -> dec_fir_async2/15(real7)
  bus_expand9/12(out5) -> dec_fir_async2/14(imag6)
  bus_expand9/11(out6) -> dec_fir_async2/13(real6)
  bus_expand9/10(out7) -> dec_fir_async2/12(imag5)
  bus_expand9/9(out8) -> dec_fir_async2/11(real5)
  bus_expand9/8(out9) -> dec_fir_async2/10(imag4)
  bus_expand9/7(out10) -> dec_fir_async2/9(real4)
  bus_expand9/6(out11) -> dec_fir_async2/8(imag3)
  bus_expand9/5(out12) -> dec_fir_async2/7(real3)
  bus_expand9/4(out13) -> dec_fir_async2/6(imag2)
  bus_expand9/3(out14) -> dec_fir_async2/5(real2)
  bus_expand9/2(out15) -> dec_fir_async2/4(imag1)
  bus_expand9/1(msb_out16) -> dec_fir_async2/3(real1)
  Delay5/1 -> bus_expand9/1(bus_in)
  bus_expand6/16(lsb_out1) -> dec_fir_async3/34(imag16)
  bus_expand6/15(out2) -> dec_fir_async3/33(real16)
  bus_expand6/13(out4) -> dec_fir_async3/31(real15)
  bus_expand6/14(out3) -> dec_fir_async3/32(imag15)
  bus_expand6/12(out5) -> dec_fir_async3/30(imag14)
  bus_expand6/11(out6) -> dec_fir_async3/29(real14)
  bus_expand6/10(out7) -> dec_fir_async3/28(imag13)
  bus_expand6/9(out8) -> dec_fir_async3/27(real13)
  bus_expand6/8(out9) -> dec_fir_async3/26(imag12)
  bus_expand6/6(out11) -> dec_fir_async3/24(imag11)
  bus_expand6/7(out10) -> dec_fir_async3/25(real12)
  bus_expand6/5(out12) -> dec_fir_async3/23(real11)
  bus_expand6/4(out13) -> dec_fir_async3/22(imag10)
  bus_expand6/2(out15) -> dec_fir_async3/20(imag9)
  bus_expand6/3(out14) -> dec_fir_async3/21(real10)
  bus_expand6/1(msb_out16) -> Gateway Out24/1, dec_fir_async3/19(real9)
  bus_expand5/16(lsb_out1) -> dec_fir_async3/18(imag8)
  bus_expand5/15(out2) -> dec_fir_async3/17(real8)
  bus_expand5/14(out3) -> dec_fir_async3/16(imag7)
  bus_expand5/13(out4) -> dec_fir_async3/15(real7)
  bus_expand5/12(out5) -> dec_fir_async3/14(imag6)
  bus_expand5/11(out6) -> dec_fir_async3/13(real6)
  bus_expand5/10(out7) -> dec_fir_async3/12(imag5)
  bus_expand5/9(out8) -> dec_fir_async3/11(real5)
  bus_expand5/8(out9) -> dec_fir_async3/10(imag4)
  bus_expand5/7(out10) -> dec_fir_async3/9(real4)
  bus_expand5/6(out11) -> dec_fir_async3/8(imag3)
  bus_expand5/5(out12) -> dec_fir_async3/7(real3)
  bus_expand5/4(out13) -> dec_fir_async3/6(imag2)
  bus_expand5/3(out14) -> dec_fir_async3/5(real2)
  bus_expand5/2(out15) -> dec_fir_async3/4(imag1)
  bus_expand5/1(msb_out16) -> Gateway Out7/1, dec_fir_async3/3(real1)
  Delay3/1 -> bus_expand5/1(bus_in)
  bus_expand3/8(out9) -> pipeline2/1(d)
  bus_expand3/6(out11) -> pipeline38/1(d)
  bus_expand3/4(out13) -> pipeline37/1(d)
  bus_expand3/2(out15) -> pipeline36/1(d)
  pipeline2/1(q) -> snap_mix_im/4(in_p0_d3)
  pipeline38/1(q) -> snap_mix_im/3(in_p0_d2)
  pipeline37/1(q) -> snap_mix_im/2(in_p0_d1)
  pipeline36/1(q) -> snap_mix_im/1(in_p0_d0)
  bus_expand1/2(lsb_out1) -> pipeline1/1(d)
  bus_expand1/1(msb_out2) -> pipeline20/1(d)
  [ddc_pol0] -> bus_expand1/1(bus_in)
  pipeline29/1(q) -> snap_ddc/5(we)
  pipeline24/1(q) -> snap_ddc/6(trig)
  pipeline26/1(q) -> snap_ddc/4(in_sync)
  pipeline25/1(q) -> snap_ddc/3(in_dv)
  pipeline1/1(q) -> snap_ddc/2(in_p0_im)
  pipeline20/1(q) -> snap_ddc/1(in_p0_re)
  bus_expand3/15(out2) -> pipeline14/1(d)
  bus_expand3/13(out4) -> pipeline13/1(d)
  bus_expand3/11(out6) -> pipeline12/1(d)
  bus_expand3/9(out8) -> pipeline11/1(d)
  bus_expand3/7(out10) -> pipeline10/1(d)
  bus_expand3/5(out12) -> pipeline9/1(d)
  bus_expand3/3(out14) -> pipeline8/1(d)
  bus_expand3/1(msb_out16) -> pipeline7/1(d)
  bus_expand4/10(out7) -> Gateway Out15/1
  bus_expand4/11(out6) -> Gateway Out16/1
  bus_expand4/12(out5) -> Gateway Out17/1
  bus_expand4/13(out4) -> Gateway Out18/1
  bus_expand4/14(out3) -> Gateway Out19/1
  bus_expand4/15(out2) -> Gateway Out20/1
  bus_expand4/16(lsb_out1) -> Gateway Out21/1
  Gateway Out21/1 -> To Workspace18/1
  Gateway Out20/1 -> To Workspace10/1
  Gateway Out19/1 -> To Workspace17/1
  Gateway Out18/1 -> To Workspace9/1
  Gateway Out17/1 -> To Workspace16/1
  Gateway Out16/1 -> To Workspace8/1
  Gateway Out15/1 -> To Workspace15/1
  bus_expand4/9(out8) -> Gateway Out14/1
  Gateway Out14/1 -> To Workspace7/1
  bus_expand4/8(out9) -> Gateway Out13/1
  Gateway Out13/1 -> To Workspace14/1
  bus_expand4/7(out10) -> Gateway Out12/1
  Gateway Out12/1 -> To Workspace6/1
  bus_expand4/6(out11) -> Gateway Out11/1
  Gateway Out11/1 -> To Workspace13/1
  bus_expand4/5(out12) -> Gateway Out10/1
  Gateway Out10/1 -> To Workspace5/1
  bus_expand4/4(out13) -> Gateway Out9/1
  Gateway Out9/1 -> To Workspace12/1
  bus_expand4/3(out14) -> Gateway Out5/1
  Gateway Out5/1 -> To Workspace4/1
  bus_expand4/2(out15) -> Gateway Out4/1
  Gateway Out4/1 -> To Workspace3/1
  bus_expand4/1(msb_out16) -> Gateway Out51/1
  [ddc_dvalid] -> ddc_dv/1
  [ddc_sync] -> pipeline21/1(d)
  pipeline22/1(q) -> Counter1/1
  pipeline21/1(q) -> Counter1/2
  Counter1/1 -> ddc_out_sync_cnt/1(out_reg)
  [cnt_rst] -> pipeline22/1(d)
  [mix_sync] -> pipeline229/1(d)
  pipeline230/1(q) -> Counter12/1
  pipeline229/1(q) -> Counter12/2
  Counter12/1 -> mix_sync_cnt/1(out_reg)
  [cnt_rst] -> pipeline230/1(d)
  [ddc_sync] -> pipeline26/1(d), pipeline24/1(d)
  [ddc_dvalid] -> pipeline25/1(d), pipeline29/1(d)
  [mix_sync] -> pipeline31/1(d), pipeline16/1(d), pipeline23/1(d), pipeline35/1(d)
  sync/1 -> Concat/1
  dvalid/1 -> Concat/2
  [mix_dvalid] -> pipeline30/1(d), pipeline15/1(d), pipeline19/1(d), pipeline34/1(d)
  [mix_80_0] -> bus_expand3/1(bus_in)
  osc1/1 -> bus_mult1/2(b)
  pol1_data/1 -> bus_mult1/1(a)
  osc0/1 -> bus_mult/2(b), bus_expand12/1(bus_in)
  pol0_data/1 -> bus_mult/1(a), bus_expand11/1(bus_in)
  [ddc_pol1] -> ddc_pol1/1
  [ddc_pol0] -> ddc_pol0/1
  [ddc_sync] -> ddc_sync/1
  Gateway Out2/1 -> To Workspace2/1
  [mix_dvalid] -> Gateway Out2/1
  [mix_sync] -> Gateway Out1/1
  Gateway Out1/1 -> To Workspace1/1
  [mix_80_0] -> bus_expand4/1(bus_in)
  Gateway Out51/1 -> To Workspace45/1
  bus_mult/2(misco) -> bus_expand/1(bus_in)
  Concat/1 -> bus_mult/3(misci)
  bus_expand/1(msb_out2) -> [mix_sync]
  From22/1 -> bus_expand2/1(bus_in)
  bus_expand7/2(lsb_out1) -> Gateway Out27/1
  bus_expand7/1(msb_out2) -> Gateway Out25/1
  Gateway Out27/1 -> To Workspace27/1
  Gateway Out25/1 -> To Workspace25/1
  bus_expand2/16(lsb_out1) -> dec_fir_async1/18(imag8)
  bus_expand2/15(out2) -> dec_fir_async1/17(real8)
  bus_expand2/14(out3) -> dec_fir_async1/16(imag7)
  bus_expand2/13(out4) -> dec_fir_async1/15(real7)
  bus_expand2/12(out5) -> dec_fir_async1/14(imag6)
  bus_expand2/11(out6) -> dec_fir_async1/13(real6)
  bus_expand2/10(out7) -> dec_fir_async1/12(imag5)
  bus_expand2/9(out8) -> dec_fir_async1/11(real5)
  bus_expand2/8(out9) -> dec_fir_async1/10(imag4)
  bus_expand2/7(out10) -> dec_fir_async1/9(real4)
  bus_expand2/6(out11) -> dec_fir_async1/8(imag3)
  bus_expand2/5(out12) -> dec_fir_async1/7(real3)
  bus_expand2/4(out13) -> dec_fir_async1/6(imag2)
  bus_expand2/3(out14) -> dec_fir_async1/5(real2)
  bus_expand2/2(out15) -> dec_fir_async1/4(imag1)
  bus_expand2/1(msb_out16) -> dec_fir_async1/3(real1)
  From24/1 -> dec_fir_async1/2(dv_in)
  From23/1 -> dec_fir_async1/1(sync_in)
